simulink model "CCMBattery"  (R2022b)
settings: Solver=FixedStepDiscrete  StartTime=0.0  StopTime=10.0  FixedStep=0.01  RelTol=1e-3
settings Model: Creator=blue.ge  LastModifiedBy=blue.ge
settings DataIOCC: SaveFormat=StructureWithTime
settings OptimizationCC: DefaultParameterBehavior=Inlined  EnhancedBackFolding=on  PassReuseOutputArgsAs=Individual arguments  ZeroExternalMemoryAtStartup=off  ZeroInternalMemoryAtStartup=off  OptimizeBlockOrder=speed  DifferentSizesBufferReuse=on  OptimizationCustomize=off  EfficientTunableParamExpr=on
settings DebuggingCC: ParameterTunabilityLossMsg=error
settings ModelReferenceCC: ModelReferenceNumInstancesAllowed=Single
settings SFSimCC: MATLABDynamicMemAlloc=off
settings RTWCC: Description=AUTOSAR  SystemTargetFile=autosar.tlc  GenCodeOnly=on  TemplateMakefile=ert_default_tmf
settings CodeAppCC: ForceParamTrailComments=on  IgnoreCustomStorageClasses=off  OperatorAnnotations=on  SimulinkDataObjDesc=on  SFDataObjDesc=on  InsertBlockDesc=on
settings STFCustomTargetCC: TargetLangStandard=C99 (ISO)  MultiwordLength=256  ParMdlRefBuildCompliant=on  CompOptLevelCompliant=on  IncludeMdlTerminateFcn=off  CombineOutputUpdateFcns=on  SuppressErrorStatus=on  ERTCustomFileBanners=on  SupportNonFinite=off  SupportComplex=off  SupportContinuousTime=off  SupportNonInlinedSFcns=off  RemoveResetFunc=on  MATLABClassNameForMDSCustomization=Simulink.SoftwareTarget.ERTCustomization  AutosarCompliant=on  UseToolchainInfoCompliant=on  LookupTableObjectStructAxisOrder=2,1,3,4,...  LUTObjectStructOrderEvenSpacing=Size,Table,Breakpoints  SystemTargetFile=autosar.tlc
settings ERTTargetCC: TargetLangStandard=C99 (ISO)  ConcurrentExecutionCompliant=off  IncludeMdlTerminateFcn=off  SuppressErrorStatus=on  SupportNonFinite=off  SupportComplex=off  ModelStepFunctionPrototypeControlCompliant=off  CPPClassGenCompliant=off  AutosarCompliant=on  LookupTableObjectStructAxisOrder=2,1,3,4,...  LUTObjectStructOrderEvenSpacing=Size,Table,Breakpoints  InlinedParameterPlacement=NonHierarchical  ERTCustomFileTemplate=example_file_process.tlc

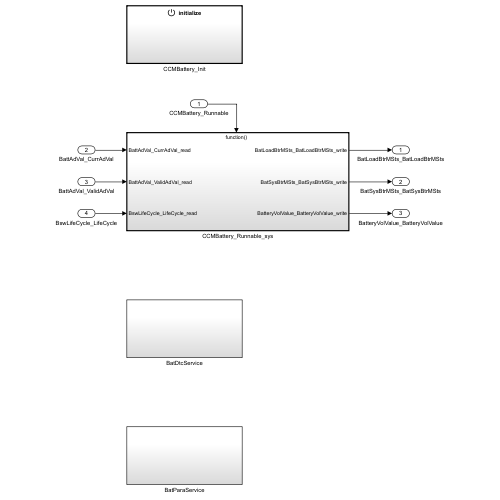
[thumbnail: Simulink's stored preview of the top-level diagram]
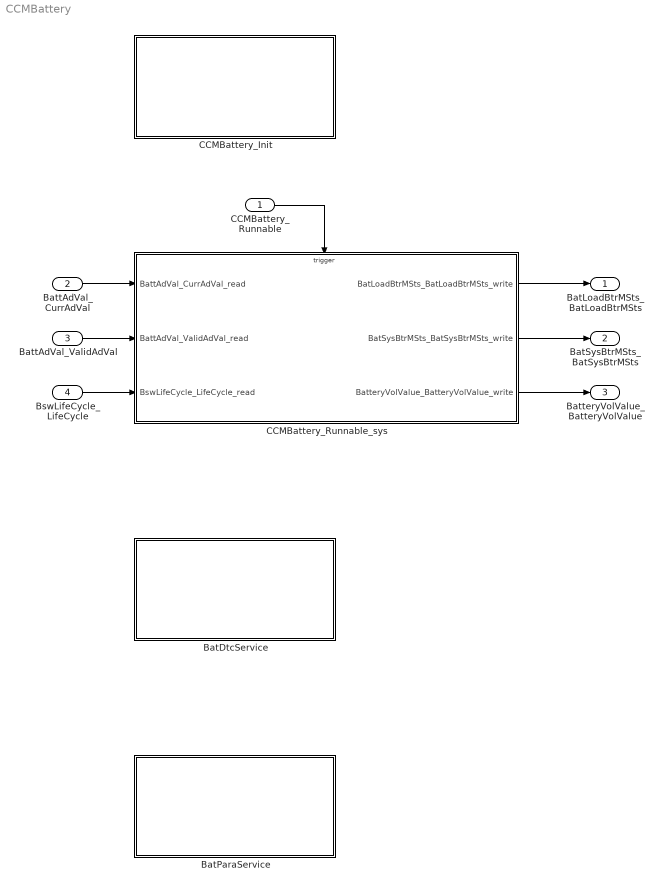
== system CCMBattery ==
  CCMBattery_Runnable : Inport  SampleTime=0.01  OutputFunctionCall=on
  BattAdVal_CurrAdVal : Inport  Port=2  OutDataTypeStr=BatCurrAd  PortDimensions=1
  BattAdVal_ValidAdVal : Inport  Port=3  OutDataTypeStr=BatValidAd  PortDimensions=1
  BswLifeCycle_LifeCycle : Inport  Port=4  OutDataTypeStr=Enum: CCMLifeCycleModeGroup  PortDimensions=1
  BatDtcService : Subsystem
  BatParaService : Subsystem
  CCMBattery_Init : Subsystem
  CCMBattery_Runnable_sys : Subsystem
  BatLoadBtrMSts_BatLoadBtrMSts : Outport  OutDataTypeStr=Enum: BatLoadBtrMStsEnum  PortDimensions=1
  BatSysBtrMSts_BatSysBtrMSts : Outport  Port=2  OutDataTypeStr=Enum: BatBtrMStsEnum  PortDimensions=1
  BatteryVolValue_BatteryVolValue : Outport  Port=3  OutDataTypeStr=BatteryVolValue  PortDimensions=1
  wires:
  BattAdVal_CurrAdVal/1 -> CCMBattery_Runnable_sys/1(BattAdVal_CurrAdVal_read)
  BattAdVal_ValidAdVal/1 -> CCMBattery_Runnable_sys/2(BattAdVal_ValidAdVal_read)
  CCMBattery_Runnable_sys/1(BatLoadBtrMSts_BatLoadBtrMSts_write) -> BatLoadBtrMSts_BatLoadBtrMSts/1
  CCMBattery_Runnable_sys/2(BatSysBtrMSts_BatSysBtrMSts_write) -> BatSysBtrMSts_BatSysBtrMSts/1
  CCMBattery_Runnable_sys/3(BatteryVolValue_BatteryVolValue_write) -> BatteryVolValue_BatteryVolValue/1
  BswLifeCycle_LifeCycle/1 -> CCMBattery_Runnable_sys/3(BswLifeCycle_LifeCycle_read)
  CCMBattery_Runnable/1 -> CCMBattery_Runnable_sys/Trigger

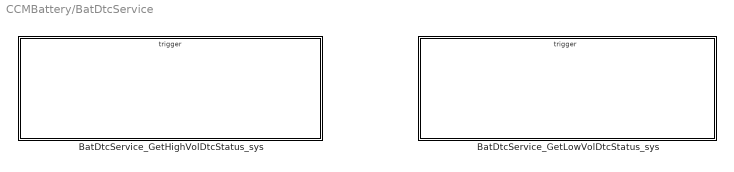
== system CCMBattery/BatDtcService ==
  BatDtcService_GetHighVolDtcStatus_sys : Subsystem
  BatDtcService_GetLowVolDtcStatus_sys : Subsystem

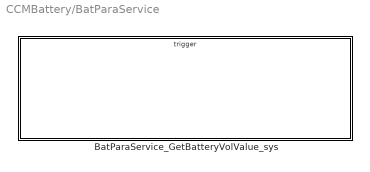
== system CCMBattery/BatParaService ==
  BatParaService_GetBatteryVolValue_sys : Subsystem

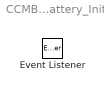
== system CCMBattery/CCMBattery_Init ==
  Event Listener : EventListener  EventName=reset

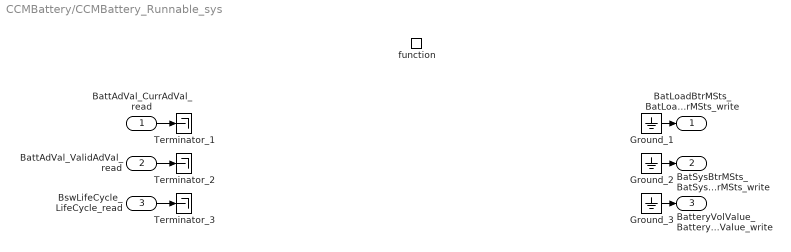
== system CCMBattery/CCMBattery_Runnable_sys ==
  BattAdVal_CurrAdVal_read : Inport
  BattAdVal_ValidAdVal_read : Inport  Port=2
  BswLifeCycle_LifeCycle_read : Inport  Port=3
  function : TriggerPort  TriggerType=function-call  VariantControl=(inherit)  SampleTimeType=periodic  SampleTime=-1
  Ground_1 : Ground
  Ground_2 : Ground
  Ground_3 : Ground
  Terminator_1 : Terminator
  Terminator_2 : Terminator
  Terminator_3 : Terminator
  BatLoadBtrMSts_BatLoadBtrMSts_write : Outport
      settings: EnsureOutportIsVirtual=on
  BatSysBtrMSts_BatSysBtrMSts_write : Outport  Port=2
      settings: EnsureOutportIsVirtual=on
  BatteryVolValue_BatteryVolValue_write : Outport  Port=3
      settings: EnsureOutportIsVirtual=on
  wires:
  BattAdVal_CurrAdVal_read/1 -> Terminator_1/1
  BattAdVal_ValidAdVal_read/1 -> Terminator_2/1
  BswLifeCycle_LifeCycle_read/1 -> Terminator_3/1
  Ground_1/1 -> BatLoadBtrMSts_BatLoadBtrMSts_write/1
  Ground_2/1 -> BatSysBtrMSts_BatSysBtrMSts_write/1
  Ground_3/1 -> BatteryVolValue_BatteryVolValue_write/1

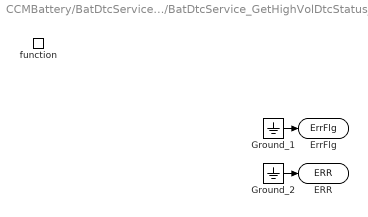
== system CCMBattery/BatDtcService/BatDtcService_GetHighVolDtcStatus_sys ==
  function : TriggerPort  TriggerType=function-call  IsSimulinkFunction=on  FunctionName=BatDtcService_GetHighVolDtcStatus  VariantControl=(inherit)
  Ground_1 : Ground
  Ground_2 : Ground
  ErrFlg : ArgOut  OutDataTypeStr=Enum: DTCState  ArgumentName=ErrFlg
  ERR : ArgOut  Port=2  OutDataTypeStr=Std_ReturnType  ArgumentName=ERR
  wires:
  Ground_1/1 -> ErrFlg/1
  Ground_2/1 -> ERR/1

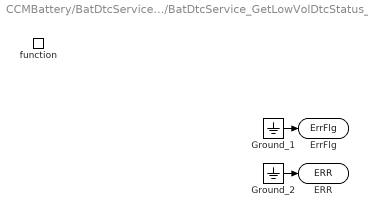
== system CCMBattery/BatDtcService/BatDtcService_GetLowVolDtcStatus_sys ==
  function : TriggerPort  TriggerType=function-call  IsSimulinkFunction=on  FunctionName=BatDtcService_GetLowVolDtcStatus  VariantControl=(inherit)
  Ground_1 : Ground
  Ground_2 : Ground
  ErrFlg : ArgOut  OutDataTypeStr=Enum: DTCState  ArgumentName=ErrFlg
  ERR : ArgOut  Port=2  OutDataTypeStr=Std_ReturnType  ArgumentName=ERR
  wires:
  Ground_1/1 -> ErrFlg/1
  Ground_2/1 -> ERR/1

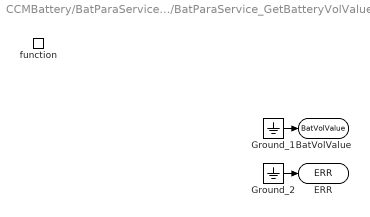
== system CCMBattery/BatParaService/BatParaService_GetBatteryVolValue_sys ==
  function : TriggerPort  TriggerType=function-call  IsSimulinkFunction=on  FunctionName=BatParaService_GetBatteryVolValue  VariantControl=(inherit)
  Ground_1 : Ground
  Ground_2 : Ground
  BatVolValue : ArgOut  OutDataTypeStr=BatteryVolValue  ArgumentName=BatVolValue
  ERR : ArgOut  Port=2  OutDataTypeStr=Std_ReturnType  ArgumentName=ERR
  wires:
  Ground_1/1 -> BatVolValue/1
  Ground_2/1 -> ERR/1
